simulink model "cddDcmApp"  (R2022b)
settings: Solver=FixedStepDiscrete  StartTime=0.0  StopTime=10.0  FixedStep=0.02  RelTol=1e-3
undefined symbols (not defined in the file or in its folder): CCPFaultType, DcChlType, HFAChlType, IcuChannelType, MtrRoutStaEnum, P_SignalPartType, P_Std_ReturnType, PowerChlType, SrsAdChlType, VGAChlType
(part 12 of 13)

== system cddDcmApp/DataServices_DID_D97B_Stepper_Motor_17_Mode_Floor_First_Right_Flap_position_DID_D97B_1_Requested_Position/DataServices_DID_D97B_Stepper_Motor_17_Mode_Floor_First_Right_Flap_position_DID_D97B_1_Requested_Position_ReadData_sys ==
  function : TriggerPort  TriggerType=function-call  IsSimulinkFunction=on  FunctionName=DataServices_DID_D97B_Stepper_Motor_17_Mode_Floor_First_Right_Flap_position_DID_D97B_1_Requested_Position_ReadData  VariantControl=(inherit)
  Ground_1 : Ground
  Ground_2 : Ground
  Data : ArgOut  OutDataTypeStr=uint8  ArgumentName=Data
  ERR : ArgOut  Port=2  OutDataTypeStr=Std_ReturnType  ArgumentName=ERR
  wires:
  Ground_1/1 -> Data/1
  Ground_2/1 -> ERR/1

== system cddDcmApp/DataServices_DID_D97B_Stepper_Motor_17_Mode_Floor_First_Right_Flap_position_DID_D97B_2_Actual_Position/DataServices_DID_D97B_Stepper_Motor_17_Mode_Floor_First_Right_Flap_position_DID_D97B_2_Actual_Position_ConditionCheckRead_sys ==
  function : TriggerPort  TriggerType=function-call  IsSimulinkFunction=on  FunctionName=<binary blob, 121 chars, not shown>  VariantControl=(inherit)
  Ground_1 : Ground
  Ground_2 : Ground
  ErrorCode : ArgOut  OutDataTypeStr=Enum: Dcm_NegativeResponseCodeType  ArgumentName=ErrorCode
  ERR : ArgOut  Port=2  OutDataTypeStr=Std_ReturnType  ArgumentName=ERR
  wires:
  Ground_1/1 -> ErrorCode/1
  Ground_2/1 -> ERR/1

== system cddDcmApp/DataServices_DID_D97B_Stepper_Motor_17_Mode_Floor_First_Right_Flap_position_DID_D97B_2_Actual_Position/DataServices_DID_D97B_Stepper_Motor_17_Mode_Floor_First_Right_Flap_position_DID_D97B_2_Actual_Position_ReadData_sys ==
  function : TriggerPort  TriggerType=function-call  IsSimulinkFunction=on  FunctionName=DataServices_DID_D97B_Stepper_Motor_17_Mode_Floor_First_Right_Flap_position_DID_D97B_2_Actual_Position_ReadData  VariantControl=(inherit)
  Ground_1 : Ground
  Ground_2 : Ground
  Data : ArgOut  OutDataTypeStr=uint8  ArgumentName=Data
  ERR : ArgOut  Port=2  OutDataTypeStr=Std_ReturnType  ArgumentName=ERR
  wires:
  Ground_1/1 -> Data/1
  Ground_2/1 -> ERR/1

== system cddDcmApp/DataServices_DID_D97C_Stepper_Motor_18_Mode_PanelFloor_Second_Left_Flap_DID_D97C_1_step_Range/DataServices_DID_D97C_Stepper_Motor_18_Mode_PanelFloor_Second_Left_Flap_DID_D97C_1_step_Range_ConditionCheckRead_sys ==
  function : TriggerPort  TriggerType=function-call  IsSimulinkFunction=on  FunctionName=DataServices_DID_D97C_Stepper_Motor_18_Mode_PanelFloor_Second_Left_Flap_DID_D97C_1_step_Range_ConditionCheckRead  VariantControl=(inherit)
  Ground_1 : Ground
  Ground_2 : Ground
  ErrorCode : ArgOut  OutDataTypeStr=Enum: Dcm_NegativeResponseCodeType  ArgumentName=ErrorCode
  ERR : ArgOut  Port=2  OutDataTypeStr=Std_ReturnType  ArgumentName=ERR
  wires:
  Ground_1/1 -> ErrorCode/1
  Ground_2/1 -> ERR/1

== system cddDcmApp/DataServices_DID_D97C_Stepper_Motor_18_Mode_PanelFloor_Second_Left_Flap_DID_D97C_1_step_Range/DataServices_DID_D97C_Stepper_Motor_18_Mode_PanelFloor_Second_Left_Flap_DID_D97C_1_step_Range_ReadData_sys ==
  function : TriggerPort  TriggerType=function-call  IsSimulinkFunction=on  FunctionName=DataServices_DID_D97C_Stepper_Motor_18_Mode_PanelFloor_Second_Left_Flap_DID_D97C_1_step_Range_ReadData  VariantControl=(inherit)
  Ground_1 : Ground
  Ground_2 : Ground
  Data : ArgOut  OutDataTypeStr=uint8  ArgumentName=Data
  ERR : ArgOut  Port=2  OutDataTypeStr=Std_ReturnType  ArgumentName=ERR
  wires:
  Ground_1/1 -> Data/1
  Ground_2/1 -> ERR/1

== system cddDcmApp/DataServices_DID_D97C_Stepper_Motor_18_Mode_PanelFloor_Second_Left_Flap_DID_D97C_1_step_Range/DataServices_DID_D97C_Stepper_Motor_18_Mode_PanelFloor_Second_Left_Flap_DID_D97C_1_step_Range_WriteData_sys ==
  function : TriggerPort  TriggerType=function-call  IsSimulinkFunction=on  FunctionName=DataServices_DID_D97C_Stepper_Motor_18_Mode_PanelFloor_Second_Left_Flap_DID_D97C_1_step_Range_WriteData  VariantControl=(inherit)
  Ground_1 : Ground
  Ground_2 : Ground
  Terminator_1 : Terminator
  Terminator_2 : Terminator
  Data : ArgIn  OutDataTypeStr=uint8  PortDimensions=2  ArgumentName=Data
  OpStatus : ArgIn  Port=2  OutDataTypeStr=Enum: Dcm_OpStatusType  ArgumentName=OpStatus
  ErrorCode : ArgOut  OutDataTypeStr=Enum: Dcm_NegativeResponseCodeType  ArgumentName=ErrorCode
  ERR : ArgOut  Port=2  OutDataTypeStr=Std_ReturnType  ArgumentName=ERR
  wires:
  Data/1 -> Terminator_1/1
  OpStatus/1 -> Terminator_2/1
  Ground_1/1 -> ErrorCode/1
  Ground_2/1 -> ERR/1

== system cddDcmApp/DataServices_DID_D97C_Stepper_Motor_18_Mode_PanelFloor_Second_Left_Flap_DID_D97C_2_No_Of_Movements/DataServices_DID_D97C_Stepper_Motor_18_Mode_PanelFloor_Second_Left_Flap_DID_D97C_2_No_Of_Movements_ConditionCheckRead_sys ==
  function : TriggerPort  TriggerType=function-call  IsSimulinkFunction=on  FunctionName=DataServices_DID_D97C_Stepper_Motor_18_Mode_PanelFloor_Second_Left_Flap_DID_D97C_2_No_Of_Movements_ConditionCheckRead  VariantControl=(inherit)
  Ground_1 : Ground
  Ground_2 : Ground
  ErrorCode : ArgOut  OutDataTypeStr=Enum: Dcm_NegativeResponseCodeType  ArgumentName=ErrorCode
  ERR : ArgOut  Port=2  OutDataTypeStr=Std_ReturnType  ArgumentName=ERR
  wires:
  Ground_1/1 -> ErrorCode/1
  Ground_2/1 -> ERR/1

== system cddDcmApp/DataServices_DID_D97C_Stepper_Motor_18_Mode_PanelFloor_Second_Left_Flap_DID_D97C_2_No_Of_Movements/DataServices_DID_D97C_Stepper_Motor_18_Mode_PanelFloor_Second_Left_Flap_DID_D97C_2_No_Of_Movements_ReadData_sys ==
  function : TriggerPort  TriggerType=function-call  IsSimulinkFunction=on  FunctionName=DataServices_DID_D97C_Stepper_Motor_18_Mode_PanelFloor_Second_Left_Flap_DID_D97C_2_No_Of_Movements_ReadData  VariantControl=(inherit)
  Ground_1 : Ground
  Ground_2 : Ground
  Data : ArgOut  OutDataTypeStr=uint8  ArgumentName=Data
  ERR : ArgOut  Port=2  OutDataTypeStr=Std_ReturnType  ArgumentName=ERR
  wires:
  Ground_1/1 -> Data/1
  Ground_2/1 -> ERR/1

== system cddDcmApp/DataServices_DID_D97C_Stepper_Motor_18_Mode_PanelFloor_Second_Left_Flap_DID_D97C_2_No_Of_Movements/DataServices_DID_D97C_Stepper_Motor_18_Mode_PanelFloor_Second_Left_Flap_DID_D97C_2_No_Of_Movements_WriteData_sys ==
  function : TriggerPort  TriggerType=function-call  IsSimulinkFunction=on  FunctionName=DataServices_DID_D97C_Stepper_Motor_18_Mode_PanelFloor_Second_Left_Flap_DID_D97C_2_No_Of_Movements_WriteData  VariantControl=(inherit)
  Ground_1 : Ground
  Ground_2 : Ground
  Terminator_1 : Terminator
  Terminator_2 : Terminator
  Data : ArgIn  OutDataTypeStr=uint8  PortDimensions=4  ArgumentName=Data
  OpStatus : ArgIn  Port=2  OutDataTypeStr=Enum: Dcm_OpStatusType  ArgumentName=OpStatus
  ErrorCode : ArgOut  OutDataTypeStr=Enum: Dcm_NegativeResponseCodeType  ArgumentName=ErrorCode
  ERR : ArgOut  Port=2  OutDataTypeStr=Std_ReturnType  ArgumentName=ERR
  wires:
  Data/1 -> Terminator_1/1
  OpStatus/1 -> Terminator_2/1
  Ground_1/1 -> ErrorCode/1
  Ground_2/1 -> ERR/1

== system cddDcmApp/DataServices_DID_D97D_Stepper_Motor_18_Mode_PanelFloor_Second_Left_Flap_position_ComplexStructure_2/DataServices_DID_D97D_Stepper_Motor_18_Mode_PanelFloor_Second_Left_Flap_position_ComplexStructure_2_ConditionCheckRead_sys ==
  function : TriggerPort  TriggerType=function-call  IsSimulinkFunction=on  FunctionName=DataServices_DID_D97D_Stepper_Motor_18_Mode_PanelFloor_Second_Left_Flap_position_ComplexStructure_2_ConditionCheckRead  VariantControl=(inherit)
  Ground_1 : Ground
  Ground_2 : Ground
  ErrorCode : ArgOut  OutDataTypeStr=Enum: Dcm_NegativeResponseCodeType  ArgumentName=ErrorCode
  ERR : ArgOut  Port=2  OutDataTypeStr=Std_ReturnType  ArgumentName=ERR
  wires:
  Ground_1/1 -> ErrorCode/1
  Ground_2/1 -> ERR/1

== system cddDcmApp/DataServices_DID_D97D_Stepper_Motor_18_Mode_PanelFloor_Second_Left_Flap_position_ComplexStructure_2/DataServices_DID_D97D_Stepper_Motor_18_Mode_PanelFloor_Second_Left_Flap_position_ComplexStructure_2_ReadData_sys ==
  function : TriggerPort  TriggerType=function-call  IsSimulinkFunction=on  FunctionName=DataServices_DID_D97D_Stepper_Motor_18_Mode_PanelFloor_Second_Left_Flap_position_ComplexStructure_2_ReadData  VariantControl=(inherit)
  Ground_1 : Ground
  Ground_2 : Ground
  Data : ArgOut  OutDataTypeStr=uint8  ArgumentName=Data
  ERR : ArgOut  Port=2  OutDataTypeStr=Std_ReturnType  ArgumentName=ERR
  wires:
  Ground_1/1 -> Data/1
  Ground_2/1 -> ERR/1

== system cddDcmApp/DataServices_DID_D97D_Stepper_Motor_18_Mode_PanelFloor_Second_Left_Flap_position_DID_D97D_1_Requested_Position/DataServices_DID_D97D_Stepper_Motor_18_Mode_PanelFloor_Second_Left_Flap_position_DID_D97D_1_Requested_Position_ReadData_sys ==
  function : TriggerPort  TriggerType=function-call  IsSimulinkFunction=on  FunctionName=DataServices_DID_D97D_Stepper_Motor_18_Mode_PanelFloor_Second_Left_Flap_position_DID_D97D_1_Requested_Position_ReadData  VariantControl=(inherit)
  Ground_1 : Ground
  Ground_2 : Ground
  Data : ArgOut  OutDataTypeStr=uint8  ArgumentName=Data
  ERR : ArgOut  Port=2  OutDataTypeStr=Std_ReturnType  ArgumentName=ERR
  wires:
  Ground_1/1 -> Data/1
  Ground_2/1 -> ERR/1

== system cddDcmApp/DataServices_DID_D97D_Stepper_Motor_18_Mode_PanelFloor_Second_Left_Flap_position_DID_D97D_2_Actual_Position/DataServices_DID_D97D_Stepper_Motor_18_Mode_PanelFloor_Second_Left_Flap_position_DID_D97D_2_Actual_Position_ConditionCheckRead_sys ==
  function : TriggerPort  TriggerType=function-call  IsSimulinkFunction=on  FunctionName=<binary blob, 126 chars, not shown>  VariantControl=(inherit)
  Ground_1 : Ground
  Ground_2 : Ground
  ErrorCode : ArgOut  OutDataTypeStr=Enum: Dcm_NegativeResponseCodeType  ArgumentName=ErrorCode
  ERR : ArgOut  Port=2  OutDataTypeStr=Std_ReturnType  ArgumentName=ERR
  wires:
  Ground_1/1 -> ErrorCode/1
  Ground_2/1 -> ERR/1

== system cddDcmApp/DataServices_DID_D97D_Stepper_Motor_18_Mode_PanelFloor_Second_Left_Flap_position_DID_D97D_2_Actual_Position/DataServices_DID_D97D_Stepper_Motor_18_Mode_PanelFloor_Second_Left_Flap_position_DID_D97D_2_Actual_Position_ReadData_sys ==
  function : TriggerPort  TriggerType=function-call  IsSimulinkFunction=on  FunctionName=DataServices_DID_D97D_Stepper_Motor_18_Mode_PanelFloor_Second_Left_Flap_position_DID_D97D_2_Actual_Position_ReadData  VariantControl=(inherit)
  Ground_1 : Ground
  Ground_2 : Ground
  Data : ArgOut  OutDataTypeStr=uint8  ArgumentName=Data
  ERR : ArgOut  Port=2  OutDataTypeStr=Std_ReturnType  ArgumentName=ERR
  wires:
  Ground_1/1 -> Data/1
  Ground_2/1 -> ERR/1

== system cddDcmApp/DataServices_DID_D97E_Stepper_Motor_19_Mode_PanelFloor_Second_Right_Flap_DID_D97E_1_step_Range/DataServices_DID_D97E_Stepper_Motor_19_Mode_PanelFloor_Second_Right_Flap_DID_D97E_1_step_Range_ConditionCheckRead_sys ==
  function : TriggerPort  TriggerType=function-call  IsSimulinkFunction=on  FunctionName=DataServices_DID_D97E_Stepper_Motor_19_Mode_PanelFloor_Second_Right_Flap_DID_D97E_1_step_Range_ConditionCheckRead  VariantControl=(inherit)
  Ground_1 : Ground
  Ground_2 : Ground
  ErrorCode : ArgOut  OutDataTypeStr=Enum: Dcm_NegativeResponseCodeType  ArgumentName=ErrorCode
  ERR : ArgOut  Port=2  OutDataTypeStr=Std_ReturnType  ArgumentName=ERR
  wires:
  Ground_1/1 -> ErrorCode/1
  Ground_2/1 -> ERR/1

== system cddDcmApp/DataServices_DID_D97E_Stepper_Motor_19_Mode_PanelFloor_Second_Right_Flap_DID_D97E_1_step_Range/DataServices_DID_D97E_Stepper_Motor_19_Mode_PanelFloor_Second_Right_Flap_DID_D97E_1_step_Range_ReadData_sys ==
  function : TriggerPort  TriggerType=function-call  IsSimulinkFunction=on  FunctionName=DataServices_DID_D97E_Stepper_Motor_19_Mode_PanelFloor_Second_Right_Flap_DID_D97E_1_step_Range_ReadData  VariantControl=(inherit)
  Ground_1 : Ground
  Ground_2 : Ground
  Data : ArgOut  OutDataTypeStr=uint8  ArgumentName=Data
  ERR : ArgOut  Port=2  OutDataTypeStr=Std_ReturnType  ArgumentName=ERR
  wires:
  Ground_1/1 -> Data/1
  Ground_2/1 -> ERR/1

== system cddDcmApp/DataServices_DID_D97E_Stepper_Motor_19_Mode_PanelFloor_Second_Right_Flap_DID_D97E_1_step_Range/DataServices_DID_D97E_Stepper_Motor_19_Mode_PanelFloor_Second_Right_Flap_DID_D97E_1_step_Range_WriteData_sys ==
  function : TriggerPort  TriggerType=function-call  IsSimulinkFunction=on  FunctionName=DataServices_DID_D97E_Stepper_Motor_19_Mode_PanelFloor_Second_Right_Flap_DID_D97E_1_step_Range_WriteData  VariantControl=(inherit)
  Ground_1 : Ground
  Ground_2 : Ground
  Terminator_1 : Terminator
  Terminator_2 : Terminator
  Data : ArgIn  OutDataTypeStr=uint8  PortDimensions=2  ArgumentName=Data
  OpStatus : ArgIn  Port=2  OutDataTypeStr=Enum: Dcm_OpStatusType  ArgumentName=OpStatus
  ErrorCode : ArgOut  OutDataTypeStr=Enum: Dcm_NegativeResponseCodeType  ArgumentName=ErrorCode
  ERR : ArgOut  Port=2  OutDataTypeStr=Std_ReturnType  ArgumentName=ERR
  wires:
  Data/1 -> Terminator_1/1
  OpStatus/1 -> Terminator_2/1
  Ground_1/1 -> ErrorCode/1
  Ground_2/1 -> ERR/1

== system cddDcmApp/DataServices_DID_D97E_Stepper_Motor_19_Mode_PanelFloor_Second_Right_Flap_DID_D97E_2_No_Of_Movements/DataServices_DID_D97E_Stepper_Motor_19_Mode_PanelFloor_Second_Right_Flap_DID_D97E_2_No_Of_Movements_ConditionCheckRead_sys ==
  function : TriggerPort  TriggerType=function-call  IsSimulinkFunction=on  FunctionName=DataServices_DID_D97E_Stepper_Motor_19_Mode_PanelFloor_Second_Right_Flap_DID_D97E_2_No_Of_Movements_ConditionCheckRead  VariantControl=(inherit)
  Ground_1 : Ground
  Ground_2 : Ground
  ErrorCode : ArgOut  OutDataTypeStr=Enum: Dcm_NegativeResponseCodeType  ArgumentName=ErrorCode
  ERR : ArgOut  Port=2  OutDataTypeStr=Std_ReturnType  ArgumentName=ERR
  wires:
  Ground_1/1 -> ErrorCode/1
  Ground_2/1 -> ERR/1

== system cddDcmApp/DataServices_DID_D97E_Stepper_Motor_19_Mode_PanelFloor_Second_Right_Flap_DID_D97E_2_No_Of_Movements/DataServices_DID_D97E_Stepper_Motor_19_Mode_PanelFloor_Second_Right_Flap_DID_D97E_2_No_Of_Movements_ReadData_sys ==
  function : TriggerPort  TriggerType=function-call  IsSimulinkFunction=on  FunctionName=DataServices_DID_D97E_Stepper_Motor_19_Mode_PanelFloor_Second_Right_Flap_DID_D97E_2_No_Of_Movements_ReadData  VariantControl=(inherit)
  Ground_1 : Ground
  Ground_2 : Ground
  Data : ArgOut  OutDataTypeStr=uint8  ArgumentName=Data
  ERR : ArgOut  Port=2  OutDataTypeStr=Std_ReturnType  ArgumentName=ERR
  wires:
  Ground_1/1 -> Data/1
  Ground_2/1 -> ERR/1

== system cddDcmApp/DataServices_DID_D97E_Stepper_Motor_19_Mode_PanelFloor_Second_Right_Flap_DID_D97E_2_No_Of_Movements/DataServices_DID_D97E_Stepper_Motor_19_Mode_PanelFloor_Second_Right_Flap_DID_D97E_2_No_Of_Movements_WriteData_sys ==
  function : TriggerPort  TriggerType=function-call  IsSimulinkFunction=on  FunctionName=DataServices_DID_D97E_Stepper_Motor_19_Mode_PanelFloor_Second_Right_Flap_DID_D97E_2_No_Of_Movements_WriteData  VariantControl=(inherit)
  Ground_1 : Ground
  Ground_2 : Ground
  Terminator_1 : Terminator
  Terminator_2 : Terminator
  Data : ArgIn  OutDataTypeStr=uint8  PortDimensions=4  ArgumentName=Data
  OpStatus : ArgIn  Port=2  OutDataTypeStr=Enum: Dcm_OpStatusType  ArgumentName=OpStatus
  ErrorCode : ArgOut  OutDataTypeStr=Enum: Dcm_NegativeResponseCodeType  ArgumentName=ErrorCode
  ERR : ArgOut  Port=2  OutDataTypeStr=Std_ReturnType  ArgumentName=ERR
  wires:
  Data/1 -> Terminator_1/1
  OpStatus/1 -> Terminator_2/1
  Ground_1/1 -> ErrorCode/1
  Ground_2/1 -> ERR/1

== system cddDcmApp/DataServices_DID_D97F_Stepper_Motor_19_Mode_PanelFloor_Second_Right_Flap_position_ComplexStructure_2/DataServices_DID_D97F_Stepper_Motor_19_Mode_PanelFloor_Second_Right_Flap_position_ComplexStructure_2_ConditionCheckRead_sys ==
  function : TriggerPort  TriggerType=function-call  IsSimulinkFunction=on  FunctionName=DataServices_DID_D97F_Stepper_Motor_19_Mode_PanelFloor_Second_Right_Flap_position_ComplexStructure_2_ConditionCheckRead  VariantControl=(inherit)
  Ground_1 : Ground
  Ground_2 : Ground
  ErrorCode : ArgOut  OutDataTypeStr=Enum: Dcm_NegativeResponseCodeType  ArgumentName=ErrorCode
  ERR : ArgOut  Port=2  OutDataTypeStr=Std_ReturnType  ArgumentName=ERR
  wires:
  Ground_1/1 -> ErrorCode/1
  Ground_2/1 -> ERR/1

== system cddDcmApp/DataServices_DID_D97F_Stepper_Motor_19_Mode_PanelFloor_Second_Right_Flap_position_ComplexStructure_2/DataServices_DID_D97F_Stepper_Motor_19_Mode_PanelFloor_Second_Right_Flap_position_ComplexStructure_2_ReadData_sys ==
  function : TriggerPort  TriggerType=function-call  IsSimulinkFunction=on  FunctionName=DataServices_DID_D97F_Stepper_Motor_19_Mode_PanelFloor_Second_Right_Flap_position_ComplexStructure_2_ReadData  VariantControl=(inherit)
  Ground_1 : Ground
  Ground_2 : Ground
  Data : ArgOut  OutDataTypeStr=uint8  ArgumentName=Data
  ERR : ArgOut  Port=2  OutDataTypeStr=Std_ReturnType  ArgumentName=ERR
  wires:
  Ground_1/1 -> Data/1
  Ground_2/1 -> ERR/1

== system cddDcmApp/DataServices_DID_D97F_Stepper_Motor_19_Mode_PanelFloor_Second_Right_Flap_position_DID_D97F_1_Requested_Position/DataServices_DID_D97F_Stepper_Motor_19_Mode_PanelFloor_Second_Right_Flap_position_DID_D97F_1_Requested_Position_ReadData_sys ==
  function : TriggerPort  TriggerType=function-call  IsSimulinkFunction=on  FunctionName=<binary blob, 120 chars, not shown>  VariantControl=(inherit)
  Ground_1 : Ground
  Ground_2 : Ground
  Data : ArgOut  OutDataTypeStr=uint8  ArgumentName=Data
  ERR : ArgOut  Port=2  OutDataTypeStr=Std_ReturnType  ArgumentName=ERR
  wires:
  Ground_1/1 -> Data/1
  Ground_2/1 -> ERR/1

== system cddDcmApp/DataServices_DID_D97F_Stepper_Motor_19_Mode_PanelFloor_Second_Right_Flap_position_DID_D97F_2_Actual_Position/DataServices_DID_D97F_Stepper_Motor_19_Mode_PanelFloor_Second_Right_Flap_position_DID_D97F_2_Actual_Position_ConditionCheckRead_sys ==
  function : TriggerPort  TriggerType=function-call  IsSimulinkFunction=on  FunctionName=<binary blob, 127 chars, not shown>  VariantControl=(inherit)
  Ground_1 : Ground
  Ground_2 : Ground
  ErrorCode : ArgOut  OutDataTypeStr=Enum: Dcm_NegativeResponseCodeType  ArgumentName=ErrorCode
  ERR : ArgOut  Port=2  OutDataTypeStr=Std_ReturnType  ArgumentName=ERR
  wires:
  Ground_1/1 -> ErrorCode/1
  Ground_2/1 -> ERR/1

== system cddDcmApp/DataServices_DID_D97F_Stepper_Motor_19_Mode_PanelFloor_Second_Right_Flap_position_DID_D97F_2_Actual_Position/DataServices_DID_D97F_Stepper_Motor_19_Mode_PanelFloor_Second_Right_Flap_position_DID_D97F_2_Actual_Position_ReadData_sys ==
  function : TriggerPort  TriggerType=function-call  IsSimulinkFunction=on  FunctionName=DataServices_DID_D97F_Stepper_Motor_19_Mode_PanelFloor_Second_Right_Flap_position_DID_D97F_2_Actual_Position_ReadData  VariantControl=(inherit)
  Ground_1 : Ground
  Ground_2 : Ground
  Data : ArgOut  OutDataTypeStr=uint8  ArgumentName=Data
  ERR : ArgOut  Port=2  OutDataTypeStr=Std_ReturnType  ArgumentName=ERR
  wires:
  Ground_1/1 -> Data/1
  Ground_2/1 -> ERR/1

== system cddDcmApp/DataServices_DID_D980_Vent_Grill_Actuator9_Second_Left_X_Flap_DID_D980/DataServices_DID_D980_Vent_Grill_Actuator9_Second_Left_X_Flap_DID_D980_ConditionCheckRead_sys ==
  function : TriggerPort  TriggerType=function-call  IsSimulinkFunction=on  FunctionName=DataServices_DID_D980_Vent_Grill_Actuator9_Second_Left_X_Flap_DID_D980_ConditionCheckRead  VariantControl=(inherit)
  Ground_1 : Ground
  Ground_2 : Ground
  ErrorCode : ArgOut  OutDataTypeStr=Enum: Dcm_NegativeResponseCodeType  ArgumentName=ErrorCode
  ERR : ArgOut  Port=2  OutDataTypeStr=Std_ReturnType  ArgumentName=ERR
  wires:
  Ground_1/1 -> ErrorCode/1
  Ground_2/1 -> ERR/1

== system cddDcmApp/DataServices_DID_D980_Vent_Grill_Actuator9_Second_Left_X_Flap_DID_D980/DataServices_DID_D980_Vent_Grill_Actuator9_Second_Left_X_Flap_DID_D980_ReadData_sys ==
  function : TriggerPort  TriggerType=function-call  IsSimulinkFunction=on  FunctionName=DataServices_DID_D980_Vent_Grill_Actuator9_Second_Left_X_Flap_DID_D980_ReadData  VariantControl=(inherit)
  Ground_1 : Ground
  Ground_2 : Ground
  Data : ArgOut  OutDataTypeStr=uint8  ArgumentName=Data
  ERR : ArgOut  Port=2  OutDataTypeStr=Std_ReturnType  ArgumentName=ERR
  wires:
  Ground_1/1 -> Data/1
  Ground_2/1 -> ERR/1

== system cddDcmApp/DataServices_DID_D980_Vent_Grill_Actuator9_Second_Left_X_Flap_DID_D980/DataServices_DID_D980_Vent_Grill_Actuator9_Second_Left_X_Flap_DID_D980_WriteData_sys ==
  function : TriggerPort  TriggerType=function-call  IsSimulinkFunction=on  FunctionName=DataServices_DID_D980_Vent_Grill_Actuator9_Second_Left_X_Flap_DID_D980_WriteData  VariantControl=(inherit)
  Ground_1 : Ground
  Ground_2 : Ground
  Terminator_1 : Terminator
  Terminator_2 : Terminator
  Data : ArgIn  OutDataTypeStr=uint8  PortDimensions=2  ArgumentName=Data
  OpStatus : ArgIn  Port=2  OutDataTypeStr=Enum: Dcm_OpStatusType  ArgumentName=OpStatus
  ErrorCode : ArgOut  OutDataTypeStr=Enum: Dcm_NegativeResponseCodeType  ArgumentName=ErrorCode
  ERR : ArgOut  Port=2  OutDataTypeStr=Std_ReturnType  ArgumentName=ERR
  wires:
  Data/1 -> Terminator_1/1
  OpStatus/1 -> Terminator_2/1
  Ground_1/1 -> ErrorCode/1
  Ground_2/1 -> ERR/1

== system cddDcmApp/DataServices_DID_D980_Vent_Grill_Actuator9_Second_Left_X_Flap_DID_D980_2_No_Of_Movements/DataServices_DID_D980_Vent_Grill_Actuator9_Second_Left_X_Flap_DID_D980_2_No_Of_Movements_ConditionCheckRead_sys ==
  function : TriggerPort  TriggerType=function-call  IsSimulinkFunction=on  FunctionName=DataServices_DID_D980_Vent_Grill_Actuator9_Second_Left_X_Flap_DID_D980_2_No_Of_Movements_ConditionCheckRead  VariantControl=(inherit)
  Ground_1 : Ground
  Ground_2 : Ground
  ErrorCode : ArgOut  OutDataTypeStr=Enum: Dcm_NegativeResponseCodeType  ArgumentName=ErrorCode
  ERR : ArgOut  Port=2  OutDataTypeStr=Std_ReturnType  ArgumentName=ERR
  wires:
  Ground_1/1 -> ErrorCode/1
  Ground_2/1 -> ERR/1

== system cddDcmApp/DataServices_DID_D980_Vent_Grill_Actuator9_Second_Left_X_Flap_DID_D980_2_No_Of_Movements/DataServices_DID_D980_Vent_Grill_Actuator9_Second_Left_X_Flap_DID_D980_2_No_Of_Movements_ReadData_sys ==
  function : TriggerPort  TriggerType=function-call  IsSimulinkFunction=on  FunctionName=DataServices_DID_D980_Vent_Grill_Actuator9_Second_Left_X_Flap_DID_D980_2_No_Of_Movements_ReadData  VariantControl=(inherit)
  Ground_1 : Ground
  Ground_2 : Ground
  Data : ArgOut  OutDataTypeStr=uint8  ArgumentName=Data
  ERR : ArgOut  Port=2  OutDataTypeStr=Std_ReturnType  ArgumentName=ERR
  wires:
  Ground_1/1 -> Data/1
  Ground_2/1 -> ERR/1

== system cddDcmApp/DataServices_DID_D980_Vent_Grill_Actuator9_Second_Left_X_Flap_DID_D980_2_No_Of_Movements/DataServices_DID_D980_Vent_Grill_Actuator9_Second_Left_X_Flap_DID_D980_2_No_Of_Movements_WriteData_sys ==
  function : TriggerPort  TriggerType=function-call  IsSimulinkFunction=on  FunctionName=DataServices_DID_D980_Vent_Grill_Actuator9_Second_Left_X_Flap_DID_D980_2_No_Of_Movements_WriteData  VariantControl=(inherit)
  Ground_1 : Ground
  Ground_2 : Ground
  Terminator_1 : Terminator
  Terminator_2 : Terminator
  Data : ArgIn  OutDataTypeStr=uint8  PortDimensions=4  ArgumentName=Data
  OpStatus : ArgIn  Port=2  OutDataTypeStr=Enum: Dcm_OpStatusType  ArgumentName=OpStatus
  ErrorCode : ArgOut  OutDataTypeStr=Enum: Dcm_NegativeResponseCodeType  ArgumentName=ErrorCode
  ERR : ArgOut  Port=2  OutDataTypeStr=Std_ReturnType  ArgumentName=ERR
  wires:
  Data/1 -> Terminator_1/1
  OpStatus/1 -> Terminator_2/1
  Ground_1/1 -> ErrorCode/1
  Ground_2/1 -> ERR/1

== system cddDcmApp/DataServices_DID_D981_Vent_Grill_Actuator9_Second_Left_X_Flap_position_ComplexStructure_2/DataServices_DID_D981_Vent_Grill_Actuator9_Second_Left_X_Flap_position_ComplexStructure_2_ConditionCheckRead_sys ==
  function : TriggerPort  TriggerType=function-call  IsSimulinkFunction=on  FunctionName=DataServices_DID_D981_Vent_Grill_Actuator9_Second_Left_X_Flap_position_ComplexStructure_2_ConditionCheckRead  VariantControl=(inherit)
  Ground_1 : Ground
  Ground_2 : Ground
  ErrorCode : ArgOut  OutDataTypeStr=Enum: Dcm_NegativeResponseCodeType  ArgumentName=ErrorCode
  ERR : ArgOut  Port=2  OutDataTypeStr=Std_ReturnType  ArgumentName=ERR
  wires:
  Ground_1/1 -> ErrorCode/1
  Ground_2/1 -> ERR/1

== system cddDcmApp/DataServices_DID_D981_Vent_Grill_Actuator9_Second_Left_X_Flap_position_ComplexStructure_2/DataServices_DID_D981_Vent_Grill_Actuator9_Second_Left_X_Flap_position_ComplexStructure_2_ReadData_sys ==
  function : TriggerPort  TriggerType=function-call  IsSimulinkFunction=on  FunctionName=DataServices_DID_D981_Vent_Grill_Actuator9_Second_Left_X_Flap_position_ComplexStructure_2_ReadData  VariantControl=(inherit)
  Ground_1 : Ground
  Ground_2 : Ground
  Data : ArgOut  OutDataTypeStr=uint8  ArgumentName=Data
  ERR : ArgOut  Port=2  OutDataTypeStr=Std_ReturnType  ArgumentName=ERR
  wires:
  Ground_1/1 -> Data/1
  Ground_2/1 -> ERR/1

== system cddDcmApp/DataServices_DID_D981_Vent_Grill_Actuator9_Second_Left_X_Flap_position_DID_D981_1_Requested_Position/DataServices_DID_D981_Vent_Grill_Actuator9_Second_Left_X_Flap_position_DID_D981_1_Requested_Position_ConditionCheckRead_sys ==
  function : TriggerPort  TriggerType=function-call  IsSimulinkFunction=on  FunctionName=DataServices_DID_D981_Vent_Grill_Actuator9_Second_Left_X_Flap_position_DID_D981_1_Requested_Position_ConditionCheckRead  VariantControl=(inherit)
  Ground_1 : Ground
  Ground_2 : Ground
  ErrorCode : ArgOut  OutDataTypeStr=Enum: Dcm_NegativeResponseCodeType  ArgumentName=ErrorCode
  ERR : ArgOut  Port=2  OutDataTypeStr=Std_ReturnType  ArgumentName=ERR
  wires:
  Ground_1/1 -> ErrorCode/1
  Ground_2/1 -> ERR/1

== system cddDcmApp/DataServices_DID_D981_Vent_Grill_Actuator9_Second_Left_X_Flap_position_DID_D981_1_Requested_Position/DataServices_DID_D981_Vent_Grill_Actuator9_Second_Left_X_Flap_position_DID_D981_1_Requested_Position_ReadData_sys ==
  function : TriggerPort  TriggerType=function-call  IsSimulinkFunction=on  FunctionName=DataServices_DID_D981_Vent_Grill_Actuator9_Second_Left_X_Flap_position_DID_D981_1_Requested_Position_ReadData  VariantControl=(inherit)
  Ground_1 : Ground
  Ground_2 : Ground
  Data : ArgOut  OutDataTypeStr=uint8  ArgumentName=Data
  ERR : ArgOut  Port=2  OutDataTypeStr=Std_ReturnType  ArgumentName=ERR
  wires:
  Ground_1/1 -> Data/1
  Ground_2/1 -> ERR/1

== system cddDcmApp/DataServices_DID_D981_Vent_Grill_Actuator9_Second_Left_X_Flap_position_DID_D981_2_Actual_Position/DataServices_DID_D981_Vent_Grill_Actuator9_Second_Left_X_Flap_position_DID_D981_2_Actual_Position_ConditionCheckRead_sys ==
  function : TriggerPort  TriggerType=function-call  IsSimulinkFunction=on  FunctionName=DataServices_DID_D981_Vent_Grill_Actuator9_Second_Left_X_Flap_position_DID_D981_2_Actual_Position_ConditionCheckRead  VariantControl=(inherit)
  Ground_1 : Ground
  Ground_2 : Ground
  ErrorCode : ArgOut  OutDataTypeStr=Enum: Dcm_NegativeResponseCodeType  ArgumentName=ErrorCode
  ERR : ArgOut  Port=2  OutDataTypeStr=Std_ReturnType  ArgumentName=ERR
  wires:
  Ground_1/1 -> ErrorCode/1
  Ground_2/1 -> ERR/1

== system cddDcmApp/DataServices_DID_D981_Vent_Grill_Actuator9_Second_Left_X_Flap_position_DID_D981_2_Actual_Position/DataServices_DID_D981_Vent_Grill_Actuator9_Second_Left_X_Flap_position_DID_D981_2_Actual_Position_ReadData_sys ==
  function : TriggerPort  TriggerType=function-call  IsSimulinkFunction=on  FunctionName=DataServices_DID_D981_Vent_Grill_Actuator9_Second_Left_X_Flap_position_DID_D981_2_Actual_Position_ReadData  VariantControl=(inherit)
  Ground_1 : Ground
  Ground_2 : Ground
  Data : ArgOut  OutDataTypeStr=uint8  ArgumentName=Data
  ERR : ArgOut  Port=2  OutDataTypeStr=Std_ReturnType  ArgumentName=ERR
  wires:
  Ground_1/1 -> Data/1
  Ground_2/1 -> ERR/1

== system cddDcmApp/DataServices_DID_D982_Vent_Grill_Actuator10_Second_Left_Y_Flap_DID_D982_1_step_Range/DataServices_DID_D982_Vent_Grill_Actuator10_Second_Left_Y_Flap_DID_D982_1_step_Range_ConditionCheckRead_sys ==
  function : TriggerPort  TriggerType=function-call  IsSimulinkFunction=on  FunctionName=DataServices_DID_D982_Vent_Grill_Actuator10_Second_Left_Y_Flap_DID_D982_1_step_Range_ConditionCheckRead  VariantControl=(inherit)
  Ground_1 : Ground
  Ground_2 : Ground
  ErrorCode : ArgOut  OutDataTypeStr=Enum: Dcm_NegativeResponseCodeType  ArgumentName=ErrorCode
  ERR : ArgOut  Port=2  OutDataTypeStr=Std_ReturnType  ArgumentName=ERR
  wires:
  Ground_1/1 -> ErrorCode/1
  Ground_2/1 -> ERR/1

== system cddDcmApp/DataServices_DID_D982_Vent_Grill_Actuator10_Second_Left_Y_Flap_DID_D982_1_step_Range/DataServices_DID_D982_Vent_Grill_Actuator10_Second_Left_Y_Flap_DID_D982_1_step_Range_ReadData_sys ==
  function : TriggerPort  TriggerType=function-call  IsSimulinkFunction=on  FunctionName=DataServices_DID_D982_Vent_Grill_Actuator10_Second_Left_Y_Flap_DID_D982_1_step_Range_ReadData  VariantControl=(inherit)
  Ground_1 : Ground
  Ground_2 : Ground
  Data : ArgOut  OutDataTypeStr=uint8  ArgumentName=Data
  ERR : ArgOut  Port=2  OutDataTypeStr=Std_ReturnType  ArgumentName=ERR
  wires:
  Ground_1/1 -> Data/1
  Ground_2/1 -> ERR/1

== system cddDcmApp/DataServices_DID_D982_Vent_Grill_Actuator10_Second_Left_Y_Flap_DID_D982_1_step_Range/DataServices_DID_D982_Vent_Grill_Actuator10_Second_Left_Y_Flap_DID_D982_1_step_Range_WriteData_sys ==
  function : TriggerPort  TriggerType=function-call  IsSimulinkFunction=on  FunctionName=DataServices_DID_D982_Vent_Grill_Actuator10_Second_Left_Y_Flap_DID_D982_1_step_Range_WriteData  VariantControl=(inherit)
  Ground_1 : Ground
  Ground_2 : Ground
  Terminator_1 : Terminator
  Terminator_2 : Terminator
  Data : ArgIn  OutDataTypeStr=uint8  PortDimensions=2  ArgumentName=Data
  OpStatus : ArgIn  Port=2  OutDataTypeStr=Enum: Dcm_OpStatusType  ArgumentName=OpStatus
  ErrorCode : ArgOut  OutDataTypeStr=Enum: Dcm_NegativeResponseCodeType  ArgumentName=ErrorCode
  ERR : ArgOut  Port=2  OutDataTypeStr=Std_ReturnType  ArgumentName=ERR
  wires:
  Data/1 -> Terminator_1/1
  OpStatus/1 -> Terminator_2/1
  Ground_1/1 -> ErrorCode/1
  Ground_2/1 -> ERR/1

== system cddDcmApp/DataServices_DID_D982_Vent_Grill_Actuator10_Second_Left_Y_Flap_DID_D982_2_No_Of_Movements/DataServices_DID_D982_Vent_Grill_Actuator10_Second_Left_Y_Flap_DID_D982_2_No_Of_Movements_ConditionCheckRead_sys ==
  function : TriggerPort  TriggerType=function-call  IsSimulinkFunction=on  FunctionName=DataServices_DID_D982_Vent_Grill_Actuator10_Second_Left_Y_Flap_DID_D982_2_No_Of_Movements_ConditionCheckRead  VariantControl=(inherit)
  Ground_1 : Ground
  Ground_2 : Ground
  ErrorCode : ArgOut  OutDataTypeStr=Enum: Dcm_NegativeResponseCodeType  ArgumentName=ErrorCode
  ERR : ArgOut  Port=2  OutDataTypeStr=Std_ReturnType  ArgumentName=ERR
  wires:
  Ground_1/1 -> ErrorCode/1
  Ground_2/1 -> ERR/1

== system cddDcmApp/DataServices_DID_D982_Vent_Grill_Actuator10_Second_Left_Y_Flap_DID_D982_2_No_Of_Movements/DataServices_DID_D982_Vent_Grill_Actuator10_Second_Left_Y_Flap_DID_D982_2_No_Of_Movements_ReadData_sys ==
  function : TriggerPort  TriggerType=function-call  IsSimulinkFunction=on  FunctionName=DataServices_DID_D982_Vent_Grill_Actuator10_Second_Left_Y_Flap_DID_D982_2_No_Of_Movements_ReadData  VariantControl=(inherit)
  Ground_1 : Ground
  Ground_2 : Ground
  Data : ArgOut  OutDataTypeStr=uint8  ArgumentName=Data
  ERR : ArgOut  Port=2  OutDataTypeStr=Std_ReturnType  ArgumentName=ERR
  wires:
  Ground_1/1 -> Data/1
  Ground_2/1 -> ERR/1

== system cddDcmApp/DataServices_DID_D982_Vent_Grill_Actuator10_Second_Left_Y_Flap_DID_D982_2_No_Of_Movements/DataServices_DID_D982_Vent_Grill_Actuator10_Second_Left_Y_Flap_DID_D982_2_No_Of_Movements_WriteData_sys ==
  function : TriggerPort  TriggerType=function-call  IsSimulinkFunction=on  FunctionName=DataServices_DID_D982_Vent_Grill_Actuator10_Second_Left_Y_Flap_DID_D982_2_No_Of_Movements_WriteData  VariantControl=(inherit)
  Ground_1 : Ground
  Ground_2 : Ground
  Terminator_1 : Terminator
  Terminator_2 : Terminator
  Data : ArgIn  OutDataTypeStr=uint8  PortDimensions=4  ArgumentName=Data
  OpStatus : ArgIn  Port=2  OutDataTypeStr=Enum: Dcm_OpStatusType  ArgumentName=OpStatus
  ErrorCode : ArgOut  OutDataTypeStr=Enum: Dcm_NegativeResponseCodeType  ArgumentName=ErrorCode
  ERR : ArgOut  Port=2  OutDataTypeStr=Std_ReturnType  ArgumentName=ERR
  wires:
  Data/1 -> Terminator_1/1
  OpStatus/1 -> Terminator_2/1
  Ground_1/1 -> ErrorCode/1
  Ground_2/1 -> ERR/1

== system cddDcmApp/DataServices_DID_D983_Vent_Grill_Actuator10_Second_Left_Y_Flap_position_ComplexStructure_2/DataServices_DID_D983_Vent_Grill_Actuator10_Second_Left_Y_Flap_position_ComplexStructure_2_ConditionCheckRead_sys ==
  function : TriggerPort  TriggerType=function-call  IsSimulinkFunction=on  FunctionName=DataServices_DID_D983_Vent_Grill_Actuator10_Second_Left_Y_Flap_position_ComplexStructure_2_ConditionCheckRead  VariantControl=(inherit)
  Ground_1 : Ground
  Ground_2 : Ground
  ErrorCode : ArgOut  OutDataTypeStr=Enum: Dcm_NegativeResponseCodeType  ArgumentName=ErrorCode
  ERR : ArgOut  Port=2  OutDataTypeStr=Std_ReturnType  ArgumentName=ERR
  wires:
  Ground_1/1 -> ErrorCode/1
  Ground_2/1 -> ERR/1

== system cddDcmApp/DataServices_DID_D983_Vent_Grill_Actuator10_Second_Left_Y_Flap_position_ComplexStructure_2/DataServices_DID_D983_Vent_Grill_Actuator10_Second_Left_Y_Flap_position_ComplexStructure_2_ReadData_sys ==
  function : TriggerPort  TriggerType=function-call  IsSimulinkFunction=on  FunctionName=DataServices_DID_D983_Vent_Grill_Actuator10_Second_Left_Y_Flap_position_ComplexStructure_2_ReadData  VariantControl=(inherit)
  Ground_1 : Ground
  Ground_2 : Ground
  Data : ArgOut  OutDataTypeStr=uint8  ArgumentName=Data
  ERR : ArgOut  Port=2  OutDataTypeStr=Std_ReturnType  ArgumentName=ERR
  wires:
  Ground_1/1 -> Data/1
  Ground_2/1 -> ERR/1

== system cddDcmApp/DataServices_DID_D983_Vent_Grill_Actuator10_Second_Left_Y_Flap_position_DID_D983_1_Requested_Position/DataServices_DID_D983_Vent_Grill_Actuator10_Second_Left_Y_Flap_position_DID_D983_1_Requested_Position_ConditionCheckRead_sys ==
  function : TriggerPort  TriggerType=function-call  IsSimulinkFunction=on  FunctionName=<binary blob, 120 chars, not shown>  VariantControl=(inherit)
  Ground_1 : Ground
  Ground_2 : Ground
  ErrorCode : ArgOut  OutDataTypeStr=Enum: Dcm_NegativeResponseCodeType  ArgumentName=ErrorCode
  ERR : ArgOut  Port=2  OutDataTypeStr=Std_ReturnType  ArgumentName=ERR
  wires:
  Ground_1/1 -> ErrorCode/1
  Ground_2/1 -> ERR/1

== system cddDcmApp/DataServices_DID_D983_Vent_Grill_Actuator10_Second_Left_Y_Flap_position_DID_D983_1_Requested_Position/DataServices_DID_D983_Vent_Grill_Actuator10_Second_Left_Y_Flap_position_DID_D983_1_Requested_Position_ReadData_sys ==
  function : TriggerPort  TriggerType=function-call  IsSimulinkFunction=on  FunctionName=DataServices_DID_D983_Vent_Grill_Actuator10_Second_Left_Y_Flap_position_DID_D983_1_Requested_Position_ReadData  VariantControl=(inherit)
  Ground_1 : Ground
  Ground_2 : Ground
  Data : ArgOut  OutDataTypeStr=uint8  ArgumentName=Data
  ERR : ArgOut  Port=2  OutDataTypeStr=Std_ReturnType  ArgumentName=ERR
  wires:
  Ground_1/1 -> Data/1
  Ground_2/1 -> ERR/1

== system cddDcmApp/DataServices_DID_D983_Vent_Grill_Actuator10_Second_Left_Y_Flap_position_DID_D983_2_Actual_Position/DataServices_DID_D983_Vent_Grill_Actuator10_Second_Left_Y_Flap_position_DID_D983_2_Actual_Position_ConditionCheckRead_sys ==
  function : TriggerPort  TriggerType=function-call  IsSimulinkFunction=on  FunctionName=DataServices_DID_D983_Vent_Grill_Actuator10_Second_Left_Y_Flap_position_DID_D983_2_Actual_Position_ConditionCheckRead  VariantControl=(inherit)
  Ground_1 : Ground
  Ground_2 : Ground
  ErrorCode : ArgOut  OutDataTypeStr=Enum: Dcm_NegativeResponseCodeType  ArgumentName=ErrorCode
  ERR : ArgOut  Port=2  OutDataTypeStr=Std_ReturnType  ArgumentName=ERR
  wires:
  Ground_1/1 -> ErrorCode/1
  Ground_2/1 -> ERR/1

== system cddDcmApp/DataServices_DID_D983_Vent_Grill_Actuator10_Second_Left_Y_Flap_position_DID_D983_2_Actual_Position/DataServices_DID_D983_Vent_Grill_Actuator10_Second_Left_Y_Flap_position_DID_D983_2_Actual_Position_ReadData_sys ==
  function : TriggerPort  TriggerType=function-call  IsSimulinkFunction=on  FunctionName=DataServices_DID_D983_Vent_Grill_Actuator10_Second_Left_Y_Flap_position_DID_D983_2_Actual_Position_ReadData  VariantControl=(inherit)
  Ground_1 : Ground
  Ground_2 : Ground
  Data : ArgOut  OutDataTypeStr=uint8  ArgumentName=Data
  ERR : ArgOut  Port=2  OutDataTypeStr=Std_ReturnType  ArgumentName=ERR
  wires:
  Ground_1/1 -> Data/1
  Ground_2/1 -> ERR/1

== system cddDcmApp/DataServices_DID_D984_Vent_Grill_Actuator11_Second_Right_X_Flap_DID_D984_1_step_Range/DataServices_DID_D984_Vent_Grill_Actuator11_Second_Right_X_Flap_DID_D984_1_step_Range_ConditionCheckRead_sys ==
  function : TriggerPort  TriggerType=function-call  IsSimulinkFunction=on  FunctionName=DataServices_DID_D984_Vent_Grill_Actuator11_Second_Right_X_Flap_DID_D984_1_step_Range_ConditionCheckRead  VariantControl=(inherit)
  Ground_1 : Ground
  Ground_2 : Ground
  ErrorCode : ArgOut  OutDataTypeStr=Enum: Dcm_NegativeResponseCodeType  ArgumentName=ErrorCode
  ERR : ArgOut  Port=2  OutDataTypeStr=Std_ReturnType  ArgumentName=ERR
  wires:
  Ground_1/1 -> ErrorCode/1
  Ground_2/1 -> ERR/1

== system cddDcmApp/DataServices_DID_D984_Vent_Grill_Actuator11_Second_Right_X_Flap_DID_D984_1_step_Range/DataServices_DID_D984_Vent_Grill_Actuator11_Second_Right_X_Flap_DID_D984_1_step_Range_ReadData_sys ==
  function : TriggerPort  TriggerType=function-call  IsSimulinkFunction=on  FunctionName=DataServices_DID_D984_Vent_Grill_Actuator11_Second_Right_X_Flap_DID_D984_1_step_Range_ReadData  VariantControl=(inherit)
  Ground_1 : Ground
  Ground_2 : Ground
  Data : ArgOut  OutDataTypeStr=uint8  ArgumentName=Data
  ERR : ArgOut  Port=2  OutDataTypeStr=Std_ReturnType  ArgumentName=ERR
  wires:
  Ground_1/1 -> Data/1
  Ground_2/1 -> ERR/1

== system cddDcmApp/DataServices_DID_D984_Vent_Grill_Actuator11_Second_Right_X_Flap_DID_D984_1_step_Range/DataServices_DID_D984_Vent_Grill_Actuator11_Second_Right_X_Flap_DID_D984_1_step_Range_WriteData_sys ==
  function : TriggerPort  TriggerType=function-call  IsSimulinkFunction=on  FunctionName=DataServices_DID_D984_Vent_Grill_Actuator11_Second_Right_X_Flap_DID_D984_1_step_Range_WriteData  VariantControl=(inherit)
  Ground_1 : Ground
  Ground_2 : Ground
  Terminator_1 : Terminator
  Terminator_2 : Terminator
  Data : ArgIn  OutDataTypeStr=uint8  PortDimensions=2  ArgumentName=Data
  OpStatus : ArgIn  Port=2  OutDataTypeStr=Enum: Dcm_OpStatusType  ArgumentName=OpStatus
  ErrorCode : ArgOut  OutDataTypeStr=Enum: Dcm_NegativeResponseCodeType  ArgumentName=ErrorCode
  ERR : ArgOut  Port=2  OutDataTypeStr=Std_ReturnType  ArgumentName=ERR
  wires:
  Data/1 -> Terminator_1/1
  OpStatus/1 -> Terminator_2/1
  Ground_1/1 -> ErrorCode/1
  Ground_2/1 -> ERR/1

== system cddDcmApp/DataServices_DID_D984_Vent_Grill_Actuator11_Second_Right_X_Flap_DID_D984_2_No_Of_Movements/DataServices_DID_D984_Vent_Grill_Actuator11_Second_Right_X_Flap_DID_D984_2_No_Of_Movements_ConditionCheckRead_sys ==
  function : TriggerPort  TriggerType=function-call  IsSimulinkFunction=on  FunctionName=DataServices_DID_D984_Vent_Grill_Actuator11_Second_Right_X_Flap_DID_D984_2_No_Of_Movements_ConditionCheckRead  VariantControl=(inherit)
  Ground_1 : Ground
  Ground_2 : Ground
  ErrorCode : ArgOut  OutDataTypeStr=Enum: Dcm_NegativeResponseCodeType  ArgumentName=ErrorCode
  ERR : ArgOut  Port=2  OutDataTypeStr=Std_ReturnType  ArgumentName=ERR
  wires:
  Ground_1/1 -> ErrorCode/1
  Ground_2/1 -> ERR/1

== system cddDcmApp/DataServices_DID_D984_Vent_Grill_Actuator11_Second_Right_X_Flap_DID_D984_2_No_Of_Movements/DataServices_DID_D984_Vent_Grill_Actuator11_Second_Right_X_Flap_DID_D984_2_No_Of_Movements_ReadData_sys ==
  function : TriggerPort  TriggerType=function-call  IsSimulinkFunction=on  FunctionName=DataServices_DID_D984_Vent_Grill_Actuator11_Second_Right_X_Flap_DID_D984_2_No_Of_Movements_ReadData  VariantControl=(inherit)
  Ground_1 : Ground
  Ground_2 : Ground
  Data : ArgOut  OutDataTypeStr=uint8  ArgumentName=Data
  ERR : ArgOut  Port=2  OutDataTypeStr=Std_ReturnType  ArgumentName=ERR
  wires:
  Ground_1/1 -> Data/1
  Ground_2/1 -> ERR/1

== system cddDcmApp/DataServices_DID_D984_Vent_Grill_Actuator11_Second_Right_X_Flap_DID_D984_2_No_Of_Movements/DataServices_DID_D984_Vent_Grill_Actuator11_Second_Right_X_Flap_DID_D984_2_No_Of_Movements_WriteData_sys ==
  function : TriggerPort  TriggerType=function-call  IsSimulinkFunction=on  FunctionName=DataServices_DID_D984_Vent_Grill_Actuator11_Second_Right_X_Flap_DID_D984_2_No_Of_Movements_WriteData  VariantControl=(inherit)
  Ground_1 : Ground
  Ground_2 : Ground
  Terminator_1 : Terminator
  Terminator_2 : Terminator
  Data : ArgIn  OutDataTypeStr=uint8  PortDimensions=4  ArgumentName=Data
  OpStatus : ArgIn  Port=2  OutDataTypeStr=Enum: Dcm_OpStatusType  ArgumentName=OpStatus
  ErrorCode : ArgOut  OutDataTypeStr=Enum: Dcm_NegativeResponseCodeType  ArgumentName=ErrorCode
  ERR : ArgOut  Port=2  OutDataTypeStr=Std_ReturnType  ArgumentName=ERR
  wires:
  Data/1 -> Terminator_1/1
  OpStatus/1 -> Terminator_2/1
  Ground_1/1 -> ErrorCode/1
  Ground_2/1 -> ERR/1

== system cddDcmApp/DataServices_DID_D985_Vent_Grill_Actuator11_Second_Right_X_Flap_position_ComplexStructure_2/DataServices_DID_D985_Vent_Grill_Actuator11_Second_Right_X_Flap_position_ComplexStructure_2_ConditionCheckRead_sys ==
  function : TriggerPort  TriggerType=function-call  IsSimulinkFunction=on  FunctionName=DataServices_DID_D985_Vent_Grill_Actuator11_Second_Right_X_Flap_position_ComplexStructure_2_ConditionCheckRead  VariantControl=(inherit)
  Ground_1 : Ground
  Ground_2 : Ground
  ErrorCode : ArgOut  OutDataTypeStr=Enum: Dcm_NegativeResponseCodeType  ArgumentName=ErrorCode
  ERR : ArgOut  Port=2  OutDataTypeStr=Std_ReturnType  ArgumentName=ERR
  wires:
  Ground_1/1 -> ErrorCode/1
  Ground_2/1 -> ERR/1

== system cddDcmApp/DataServices_DID_D985_Vent_Grill_Actuator11_Second_Right_X_Flap_position_ComplexStructure_2/DataServices_DID_D985_Vent_Grill_Actuator11_Second_Right_X_Flap_position_ComplexStructure_2_ReadData_sys ==
  function : TriggerPort  TriggerType=function-call  IsSimulinkFunction=on  FunctionName=DataServices_DID_D985_Vent_Grill_Actuator11_Second_Right_X_Flap_position_ComplexStructure_2_ReadData  VariantControl=(inherit)
  Ground_1 : Ground
  Ground_2 : Ground
  Data : ArgOut  OutDataTypeStr=uint8  ArgumentName=Data
  ERR : ArgOut  Port=2  OutDataTypeStr=Std_ReturnType  ArgumentName=ERR
  wires:
  Ground_1/1 -> Data/1
  Ground_2/1 -> ERR/1

== system cddDcmApp/DataServices_DID_D985_Vent_Grill_Actuator11_Second_Right_X_Flap_position_DID_D985_1_Requested_Position/DataServices_DID_D985_Vent_Grill_Actuator11_Second_Right_X_Flap_position_DID_D985_1_Requested_Position_ConditionCheckRead_sys ==
  function : TriggerPort  TriggerType=function-call  IsSimulinkFunction=on  FunctionName=<binary blob, 121 chars, not shown>  VariantControl=(inherit)
  Ground_1 : Ground
  Ground_2 : Ground
  ErrorCode : ArgOut  OutDataTypeStr=Enum: Dcm_NegativeResponseCodeType  ArgumentName=ErrorCode
  ERR : ArgOut  Port=2  OutDataTypeStr=Std_ReturnType  ArgumentName=ERR
  wires:
  Ground_1/1 -> ErrorCode/1
  Ground_2/1 -> ERR/1

== system cddDcmApp/DataServices_DID_D985_Vent_Grill_Actuator11_Second_Right_X_Flap_position_DID_D985_1_Requested_Position/DataServices_DID_D985_Vent_Grill_Actuator11_Second_Right_X_Flap_position_DID_D985_1_Requested_Position_ReadData_sys ==
  function : TriggerPort  TriggerType=function-call  IsSimulinkFunction=on  FunctionName=DataServices_DID_D985_Vent_Grill_Actuator11_Second_Right_X_Flap_position_DID_D985_1_Requested_Position_ReadData  VariantControl=(inherit)
  Ground_1 : Ground
  Ground_2 : Ground
  Data : ArgOut  OutDataTypeStr=uint8  ArgumentName=Data
  ERR : ArgOut  Port=2  OutDataTypeStr=Std_ReturnType  ArgumentName=ERR
  wires:
  Ground_1/1 -> Data/1
  Ground_2/1 -> ERR/1

== system cddDcmApp/DataServices_DID_D985_Vent_Grill_Actuator11_Second_Right_X_Flap_position_DID_D985_2_Actual_Position/DataServices_DID_D985_Vent_Grill_Actuator11_Second_Right_X_Flap_position_DID_D985_2_Actual_Position_ConditionCheckRead_sys ==
  function : TriggerPort  TriggerType=function-call  IsSimulinkFunction=on  FunctionName=DataServices_DID_D985_Vent_Grill_Actuator11_Second_Right_X_Flap_position_DID_D985_2_Actual_Position_ConditionCheckRead  VariantControl=(inherit)
  Ground_1 : Ground
  Ground_2 : Ground
  ErrorCode : ArgOut  OutDataTypeStr=Enum: Dcm_NegativeResponseCodeType  ArgumentName=ErrorCode
  ERR : ArgOut  Port=2  OutDataTypeStr=Std_ReturnType  ArgumentName=ERR
  wires:
  Ground_1/1 -> ErrorCode/1
  Ground_2/1 -> ERR/1

== system cddDcmApp/DataServices_DID_D985_Vent_Grill_Actuator11_Second_Right_X_Flap_position_DID_D985_2_Actual_Position/DataServices_DID_D985_Vent_Grill_Actuator11_Second_Right_X_Flap_position_DID_D985_2_Actual_Position_ReadData_sys ==
  function : TriggerPort  TriggerType=function-call  IsSimulinkFunction=on  FunctionName=DataServices_DID_D985_Vent_Grill_Actuator11_Second_Right_X_Flap_position_DID_D985_2_Actual_Position_ReadData  VariantControl=(inherit)
  Ground_1 : Ground
  Ground_2 : Ground
  Data : ArgOut  OutDataTypeStr=uint8  ArgumentName=Data
  ERR : ArgOut  Port=2  OutDataTypeStr=Std_ReturnType  ArgumentName=ERR
  wires:
  Ground_1/1 -> Data/1
  Ground_2/1 -> ERR/1

== system cddDcmApp/DataServices_DID_D986_Vent_Grill_Actuator12_Second_Right_Y_Flap_DID_D986_1_step_Range/DataServices_DID_D986_Vent_Grill_Actuator12_Second_Right_Y_Flap_DID_D986_1_step_Range_ConditionCheckRead_sys ==
  function : TriggerPort  TriggerType=function-call  IsSimulinkFunction=on  FunctionName=DataServices_DID_D986_Vent_Grill_Actuator12_Second_Right_Y_Flap_DID_D986_1_step_Range_ConditionCheckRead  VariantControl=(inherit)
  Ground_1 : Ground
  Ground_2 : Ground
  ErrorCode : ArgOut  OutDataTypeStr=Enum: Dcm_NegativeResponseCodeType  ArgumentName=ErrorCode
  ERR : ArgOut  Port=2  OutDataTypeStr=Std_ReturnType  ArgumentName=ERR
  wires:
  Ground_1/1 -> ErrorCode/1
  Ground_2/1 -> ERR/1

== system cddDcmApp/DataServices_DID_D986_Vent_Grill_Actuator12_Second_Right_Y_Flap_DID_D986_1_step_Range/DataServices_DID_D986_Vent_Grill_Actuator12_Second_Right_Y_Flap_DID_D986_1_step_Range_ReadData_sys ==
  function : TriggerPort  TriggerType=function-call  IsSimulinkFunction=on  FunctionName=DataServices_DID_D986_Vent_Grill_Actuator12_Second_Right_Y_Flap_DID_D986_1_step_Range_ReadData  VariantControl=(inherit)
  Ground_1 : Ground
  Ground_2 : Ground
  Data : ArgOut  OutDataTypeStr=uint8  ArgumentName=Data
  ERR : ArgOut  Port=2  OutDataTypeStr=Std_ReturnType  ArgumentName=ERR
  wires:
  Ground_1/1 -> Data/1
  Ground_2/1 -> ERR/1

== system cddDcmApp/DataServices_DID_D986_Vent_Grill_Actuator12_Second_Right_Y_Flap_DID_D986_1_step_Range/DataServices_DID_D986_Vent_Grill_Actuator12_Second_Right_Y_Flap_DID_D986_1_step_Range_WriteData_sys ==
  function : TriggerPort  TriggerType=function-call  IsSimulinkFunction=on  FunctionName=DataServices_DID_D986_Vent_Grill_Actuator12_Second_Right_Y_Flap_DID_D986_1_step_Range_WriteData  VariantControl=(inherit)
  Ground_1 : Ground
  Ground_2 : Ground
  Terminator_1 : Terminator
  Terminator_2 : Terminator
  Data : ArgIn  OutDataTypeStr=uint8  PortDimensions=2  ArgumentName=Data
  OpStatus : ArgIn  Port=2  OutDataTypeStr=Enum: Dcm_OpStatusType  ArgumentName=OpStatus
  ErrorCode : ArgOut  OutDataTypeStr=Enum: Dcm_NegativeResponseCodeType  ArgumentName=ErrorCode
  ERR : ArgOut  Port=2  OutDataTypeStr=Std_ReturnType  ArgumentName=ERR
  wires:
  Data/1 -> Terminator_1/1
  OpStatus/1 -> Terminator_2/1
  Ground_1/1 -> ErrorCode/1
  Ground_2/1 -> ERR/1

== system cddDcmApp/DataServices_DID_D986_Vent_Grill_Actuator12_Second_Right_Y_Flap_DID_D986_2_No_Of_Movements/DataServices_DID_D986_Vent_Grill_Actuator12_Second_Right_Y_Flap_DID_D986_2_No_Of_Movements_ConditionCheckRead_sys ==
  function : TriggerPort  TriggerType=function-call  IsSimulinkFunction=on  FunctionName=DataServices_DID_D986_Vent_Grill_Actuator12_Second_Right_Y_Flap_DID_D986_2_No_Of_Movements_ConditionCheckRead  VariantControl=(inherit)
  Ground_1 : Ground
  Ground_2 : Ground
  ErrorCode : ArgOut  OutDataTypeStr=Enum: Dcm_NegativeResponseCodeType  ArgumentName=ErrorCode
  ERR : ArgOut  Port=2  OutDataTypeStr=Std_ReturnType  ArgumentName=ERR
  wires:
  Ground_1/1 -> ErrorCode/1
  Ground_2/1 -> ERR/1

== system cddDcmApp/DataServices_DID_D986_Vent_Grill_Actuator12_Second_Right_Y_Flap_DID_D986_2_No_Of_Movements/DataServices_DID_D986_Vent_Grill_Actuator12_Second_Right_Y_Flap_DID_D986_2_No_Of_Movements_ReadData_sys ==
  function : TriggerPort  TriggerType=function-call  IsSimulinkFunction=on  FunctionName=DataServices_DID_D986_Vent_Grill_Actuator12_Second_Right_Y_Flap_DID_D986_2_No_Of_Movements_ReadData  VariantControl=(inherit)
  Ground_1 : Ground
  Ground_2 : Ground
  Data : ArgOut  OutDataTypeStr=uint8  ArgumentName=Data
  ERR : ArgOut  Port=2  OutDataTypeStr=Std_ReturnType  ArgumentName=ERR
  wires:
  Ground_1/1 -> Data/1
  Ground_2/1 -> ERR/1
